simulink model "Demo_TrafficFlow_cs"  (Simulink 7.6) (continued)
== system Demo_TrafficFlow_cs == (continued)
      mask Display: image(imread('ico_OBU_RF_24_gray.png'))
  RFOBU_10_Tx : Subsystem  Tag=OBUTx  Priority=0  mask(PreScan Sink Block: AntennaT){PortName=RFOBU_10, PortIndex=201, PortWidth=16, TimeStep=1/20}
      mask prompts: PortName "Auto Assigned: Port Name [-]"; PortIndex "Auto Assigned: Index [-]"; PortWidth "Auto Assigned: Port Dimensions [-]"; TimeStep "Auto Assigned: Upload Period [s]"
      mask help:
      | Using the Beacon and OBU (On-Board Unit) information between actors and infrastructure can be exchanged.
      |
      | The OBU Transmitter contains the following, user-definable signals:
      |
      | - Vehicle Type		[-]
      | - Spares (1:15)		[-]
      |
      | For more information, please click Help.
      settings: DeleteFcn=PreScanBlockDeleteFcn;
      mask Help: eval('openHelp(487)')
      mask Display: image(imread('ico_OBU_RF_24_gray.png'))
  RFOBU_1_Rx : Subsystem  Tag=OBURx  Priority=3  mask(PreScan Source Block: Antenna Receiver){NrEntitiesOut=2, PortName=RFOBU_1, PortIndex=8, PortWidth=40, TimeStep=1/20}
      mask prompts: NrEntitiesOut "User Specified: # of Visible OBUs"; PortName "Auto Assigned: Port Name [-]"; PortIndex "Auto Assigned: Index [-]"; PortWidth "Auto Assigned: Port Dimensions [-]"; TimeStep "Auto Assigned: Upload Period [s]"
      mask help:
      | Using the Beacon and OBU (On-Board Unit) information between actors and infrastructure can be exchanged.
      |
      | The OBU Reciever receives infrastructure information and user-definable signals.
      |
      | For more information, please click Help.
      settings: DeleteFcn=PreScanBlockDeleteFcn;
      mask Help: eval('openHelp(487)')
      mask Display: image(imread('ico_OBU_RF_24_gray.png'))
  RFOBU_1_Tx : Subsystem  Tag=OBUTx  Priority=0  mask(PreScan Sink Block: AntennaT){PortName=RFOBU_1, PortIndex=15, PortWidth=16, TimeStep=1/20}
      mask prompts: PortName "Auto Assigned: Port Name [-]"; PortIndex "Auto Assigned: Index [-]"; PortWidth "Auto Assigned: Port Dimensions [-]"; TimeStep "Auto Assigned: Upload Period [s]"
      mask help:
      | Using the Beacon and OBU (On-Board Unit) information between actors and infrastructure can be exchanged.
      |
      | The OBU Transmitter contains the following, user-definable signals:
      |
      | - Vehicle Type		[-]
      | - Spares (1:15)		[-]
      |
      | For more information, please click Help.
      settings: DeleteFcn=PreScanBlockDeleteFcn;
      mask Help: eval('openHelp(487)')
      mask Display: image(imread('ico_OBU_RF_24_gray.png'))
  RFOBU_2_Rx : Subsystem  Tag=OBURx  Priority=3  mask(PreScan Source Block: Antenna Receiver){NrEntitiesOut=2, PortName=RFOBU_2, PortIndex=116, PortWidth=40, TimeStep=1/20}
      mask prompts: NrEntitiesOut "User Specified: # of Visible OBUs"; PortName "Auto Assigned: Port Name [-]"; PortIndex "Auto Assigned: Index [-]"; PortWidth "Auto Assigned: Port Dimensions [-]"; TimeStep "Auto Assigned: Upload Period [s]"
      mask help:
      | Using the Beacon and OBU (On-Board Unit) information between actors and infrastructure can be exchanged.
      |
      | The OBU Reciever receives infrastructure information and user-definable signals.
      |
      | For more information, please click Help.
      settings: DeleteFcn=PreScanBlockDeleteFcn;
      mask Help: eval('openHelp(487)')
      mask Display: image(imread('ico_OBU_RF_24_gray.png'))
  RFOBU_2_Tx : Subsystem  Tag=OBUTx  Priority=0  mask(PreScan Sink Block: AntennaT){PortName=RFOBU_2, PortIndex=46, PortWidth=16, TimeStep=1/20}
      mask prompts: PortName "Auto Assigned: Port Name [-]"; PortIndex "Auto Assigned: Index [-]"; PortWidth "Auto Assigned: Port Dimensions [-]"; TimeStep "Auto Assigned: Upload Period [s]"
      mask help:
      | Using the Beacon and OBU (On-Board Unit) information between actors and infrastructure can be exchanged.
      |
      | The OBU Transmitter contains the following, user-definable signals:
      |
      | - Vehicle Type		[-]
      | - Spares (1:15)		[-]
      |
      | For more information, please click Help.
      settings: DeleteFcn=PreScanBlockDeleteFcn;
      mask Help: eval('openHelp(487)')
      mask Display: image(imread('ico_OBU_RF_24_gray.png'))
  RFOBU_3_Rx : Subsystem  Tag=OBURx  Priority=3  mask(PreScan Source Block: Antenna Receiver){NrEntitiesOut=2, PortName=RFOBU_3, PortIndex=224, PortWidth=40, TimeStep=1/20}
      mask prompts: NrEntitiesOut "User Specified: # of Visible OBUs"; PortName "Auto Assigned: Port Name [-]"; PortIndex "Auto Assigned: Index [-]"; PortWidth "Auto Assigned: Port Dimensions [-]"; TimeStep "Auto Assigned: Upload Period [s]"
      mask help:
      | Using the Beacon and OBU (On-Board Unit) information between actors and infrastructure can be exchanged.
      |
      | The OBU Reciever receives infrastructure information and user-definable signals.
      |
      | For more information, please click Help.
      settings: DeleteFcn=PreScanBlockDeleteFcn;
      mask Help: eval('openHelp(487)')
      mask Display: image(imread('ico_OBU_RF_24_gray.png'))
  RFOBU_3_Tx : Subsystem  Tag=OBUTx  Priority=0  mask(PreScan Sink Block: AntennaT){PortName=RFOBU_3, PortIndex=77, PortWidth=16, TimeStep=1/20}
      mask prompts: PortName "Auto Assigned: Port Name [-]"; PortIndex "Auto Assigned: Index [-]"; PortWidth "Auto Assigned: Port Dimensions [-]"; TimeStep "Auto Assigned: Upload Period [s]"
      mask help:
      | Using the Beacon and OBU (On-Board Unit) information between actors and infrastructure can be exchanged.
      |
      | The OBU Transmitter contains the following, user-definable signals:
      |
      | - Vehicle Type		[-]
      | - Spares (1:15)		[-]
      |
      | For more information, please click Help.
      settings: DeleteFcn=PreScanBlockDeleteFcn;
      mask Help: eval('openHelp(487)')
      mask Display: image(imread('ico_OBU_RF_24_gray.png'))
  RFOBU_4_Rx : Subsystem  Tag=OBURx  Priority=3  mask(PreScan Source Block: Antenna Receiver){NrEntitiesOut=2, PortName=RFOBU_4, PortIndex=332, PortWidth=40, TimeStep=1/20}
      mask prompts: NrEntitiesOut "User Specified: # of Visible OBUs"; PortName "Auto Assigned: Port Name [-]"; PortIndex "Auto Assigned: Index [-]"; PortWidth "Auto Assigned: Port Dimensions [-]"; TimeStep "Auto Assigned: Upload Period [s]"
      mask help:
      | Using the Beacon and OBU (On-Board Unit) information between actors and infrastructure can be exchanged.
      |
      | The OBU Reciever receives infrastructure information and user-definable signals.
      |
      | For more information, please click Help.
      settings: DeleteFcn=PreScanBlockDeleteFcn;
      mask Help: eval('openHelp(487)')
      mask Display: image(imread('ico_OBU_RF_24_gray.png'))
  RFOBU_4_Tx : Subsystem  Tag=OBUTx  Priority=0  mask(PreScan Sink Block: AntennaT){PortName=RFOBU_4, PortIndex=108, PortWidth=16, TimeStep=1/20}
      mask prompts: PortName "Auto Assigned: Port Name [-]"; PortIndex "Auto Assigned: Index [-]"; PortWidth "Auto Assigned: Port Dimensions [-]"; TimeStep "Auto Assigned: Upload Period [s]"
      mask help:
      | Using the Beacon and OBU (On-Board Unit) information between actors and infrastructure can be exchanged.
      |
      | The OBU Transmitter contains the following, user-definable signals:
      |
      | - Vehicle Type		[-]
      | - Spares (1:15)		[-]
      |
      | For more information, please click Help.
      settings: DeleteFcn=PreScanBlockDeleteFcn;
      mask Help: eval('openHelp(487)')
      mask Display: image(imread('ico_OBU_RF_24_gray.png'))
  RFOBU_5_Rx : Subsystem  Tag=OBURx  Priority=3  mask(PreScan Source Block: Antenna Receiver){NrEntitiesOut=2, PortName=RFOBU_5, PortIndex=440, PortWidth=40, TimeStep=1/20}
      mask prompts: NrEntitiesOut "User Specified: # of Visible OBUs"; PortName "Auto Assigned: Port Name [-]"; PortIndex "Auto Assigned: Index [-]"; PortWidth "Auto Assigned: Port Dimensions [-]"; TimeStep "Auto Assigned: Upload Period [s]"
      mask help:
      | Using the Beacon and OBU (On-Board Unit) information between actors and infrastructure can be exchanged.
      |
      | The OBU Reciever receives infrastructure information and user-definable signals.
      |
      | For more information, please click Help.
      settings: DeleteFcn=PreScanBlockDeleteFcn;
      mask Help: eval('openHelp(487)')
      mask Display: image(imread('ico_OBU_RF_24_gray.png'))
  RFOBU_5_Tx : Subsystem  Tag=OBUTx  Priority=0  mask(PreScan Sink Block: AntennaT){PortName=RFOBU_5, PortIndex=139, PortWidth=16, TimeStep=1/20}
      mask prompts: PortName "Auto Assigned: Port Name [-]"; PortIndex "Auto Assigned: Index [-]"; PortWidth "Auto Assigned: Port Dimensions [-]"; TimeStep "Auto Assigned: Upload Period [s]"
      mask help:
      | Using the Beacon and OBU (On-Board Unit) information between actors and infrastructure can be exchanged.
      |
      | The OBU Transmitter contains the following, user-definable signals:
      |
      | - Vehicle Type		[-]
      | - Spares (1:15)		[-]
      |
      | For more information, please click Help.
      settings: DeleteFcn=PreScanBlockDeleteFcn;
      mask Help: eval('openHelp(487)')
      mask Display: image(imread('ico_OBU_RF_24_gray.png'))
  RFOBU_6_Rx : Subsystem  Tag=OBURx  Priority=3  mask(PreScan Source Block: Antenna Receiver){NrEntitiesOut=2, PortName=RFOBU_6, PortIndex=548, PortWidth=40, TimeStep=1/20}
      mask prompts: NrEntitiesOut "User Specified: # of Visible OBUs"; PortName "Auto Assigned: Port Name [-]"; PortIndex "Auto Assigned: Index [-]"; PortWidth "Auto Assigned: Port Dimensions [-]"; TimeStep "Auto Assigned: Upload Period [s]"
      mask help:
      | Using the Beacon and OBU (On-Board Unit) information between actors and infrastructure can be exchanged.
      |
      | The OBU Reciever receives infrastructure information and user-definable signals.
      |
      | For more information, please click Help.
      settings: DeleteFcn=PreScanBlockDeleteFcn;
      mask Help: eval('openHelp(487)')
      mask Display: image(imread('ico_OBU_RF_24_gray.png'))
  RFOBU_6_Tx : Subsystem  Tag=OBUTx  Priority=0  mask(PreScan Sink Block: AntennaT){PortName=RFOBU_6, PortIndex=170, PortWidth=16, TimeStep=1/20}
      mask prompts: PortName "Auto Assigned: Port Name [-]"; PortIndex "Auto Assigned: Index [-]"; PortWidth "Auto Assigned: Port Dimensions [-]"; TimeStep "Auto Assigned: Upload Period [s]"
      mask help:
      | Using the Beacon and OBU (On-Board Unit) information between actors and infrastructure can be exchanged.
      |
      | The OBU Transmitter contains the following, user-definable signals:
      |
      | - Vehicle Type		[-]
      | - Spares (1:15)		[-]
      |
      | For more information, please click Help.
      settings: DeleteFcn=PreScanBlockDeleteFcn;
      mask Help: eval('openHelp(487)')
      mask Display: image(imread('ico_OBU_RF_24_gray.png'))
  RFOBU_7_Rx : Subsystem  Tag=OBURx  Priority=3  mask(PreScan Source Block: Antenna Receiver){NrEntitiesOut=2, PortName=RFOBU_7, PortIndex=764, PortWidth=40, TimeStep=1/20}
      mask prompts: NrEntitiesOut "User Specified: # of Visible OBUs"; PortName "Auto Assigned: Port Name [-]"; PortIndex "Auto Assigned: Index [-]"; PortWidth "Auto Assigned: Port Dimensions [-]"; TimeStep "Auto Assigned: Upload Period [s]"
      mask help:
      | Using the Beacon and OBU (On-Board Unit) information between actors and infrastructure can be exchanged.
      |
      | The OBU Reciever receives infrastructure information and user-definable signals.
      |
      | For more information, please click Help.
      settings: DeleteFcn=PreScanBlockDeleteFcn;
      mask Help: eval('openHelp(487)')
      mask Display: image(imread('ico_OBU_RF_24_gray.png'))
  RFOBU_7_Tx : Subsystem  Tag=OBUTx  Priority=0  mask(PreScan Sink Block: AntennaT){PortName=RFOBU_7, PortIndex=232, PortWidth=16, TimeStep=1/20}
      mask prompts: PortName "Auto Assigned: Port Name [-]"; PortIndex "Auto Assigned: Index [-]"; PortWidth "Auto Assigned: Port Dimensions [-]"; TimeStep "Auto Assigned: Upload Period [s]"
      mask help:
      | Using the Beacon and OBU (On-Board Unit) information between actors and infrastructure can be exchanged.
      |
      | The OBU Transmitter contains the following, user-definable signals:
      |
      | - Vehicle Type		[-]
      | - Spares (1:15)		[-]
      |
      | For more information, please click Help.
      settings: DeleteFcn=PreScanBlockDeleteFcn;
      mask Help: eval('openHelp(487)')
      mask Display: image(imread('ico_OBU_RF_24_gray.png'))
  SELF_Audi_A8_1 : Subsystem  Tag=SELF  Priority=3  mask(PreScan Source Block: SELF){PortName=SELF_Audi_A8_1, PortIndex=0, PortWidth=8, TimeStep=1/20}
      mask prompts: PortName "Auto-Assigned: Port Name [-]"; PortIndex "Auto-Assigned: Port Index [-]"; PortWidth "Auto-Assigned: Port Dimensions"; TimeStep "Auto-Assigned: Upload Period [s]"
      mask help:
      | The SELF port shows (state) information of the actor itself. The outputs are position in the experiment's axis system and the GPS coordinates, and the object's absolute velocity and compass heading.
      |
      | For more information, please click Help.
      settings: DeleteFcn=PreScanBlockDeleteFcn;
      mask Help: eval('openHelp(462)')
      mask Display: image(imread('GPS.png'))
  SELF_BMW_X5_1 : Subsystem  Tag=SELF  Priority=3  mask(PreScan Source Block: SELF){PortName=SELF_BMW_X5_1, PortIndex=108, PortWidth=8, TimeStep=1/20}
      mask prompts: PortName "Auto-Assigned: Port Name [-]"; PortIndex "Auto-Assigned: Port Index [-]"; PortWidth "Auto-Assigned: Port Dimensions"; TimeStep "Auto-Assigned: Upload Period [s]"
      mask help:
      | The SELF port shows (state) information of the actor itself. The outputs are position in the experiment's axis system and the GPS coordinates, and the object's absolute velocity and compass heading.
      |
      | For more information, please click Help.
      settings: DeleteFcn=PreScanBlockDeleteFcn;
      mask Help: eval('openHelp(462)')
      mask Display: image(imread('GPS.png'))
  SELF_Citroen_C3_1 : Subsystem  Tag=SELF  Priority=3  mask(PreScan Source Block: SELF){PortName=SELF_Citroen_C3_1, PortIndex=216, PortWidth=8, TimeStep=1/20}
      mask prompts: PortName "Auto-Assigned: Port Name [-]"; PortIndex "Auto-Assigned: Port Index [-]"; PortWidth "Auto-Assigned: Port Dimensions"; TimeStep "Auto-Assigned: Upload Period [s]"
      mask help:
      | The SELF port shows (state) information of the actor itself. The outputs are position in the experiment's axis system and the GPS coordinates, and the object's absolute velocity and compass heading.
      |
      | For more information, please click Help.
      settings: DeleteFcn=PreScanBlockDeleteFcn;
      mask Help: eval('openHelp(462)')
      mask Display: image(imread('GPS.png'))
  SELF_Mazda_RX8_1 : Subsystem  Tag=SELF  Priority=3  mask(PreScan Source Block: SELF){PortName=SELF_Mazda_RX8_1, PortIndex=324, PortWidth=8, TimeStep=1/20}
      mask prompts: PortName "Auto-Assigned: Port Name [-]"; PortIndex "Auto-Assigned: Port Index [-]"; PortWidth "Auto-Assigned: Port Dimensions"; TimeStep "Auto-Assigned: Upload Period [s]"
      mask help:
      | The SELF port shows (state) information of the actor itself. The outputs are position in the experiment's axis system and the GPS coordinates, and the object's absolute velocity and compass heading.
      |
      | For more information, please click Help.
      settings: DeleteFcn=PreScanBlockDeleteFcn;
      mask Help: eval('openHelp(462)')
      mask Display: image(imread('GPS.png'))
  SELF_Motorcyclist_1 : Subsystem  Tag=SELF  Priority=3  mask(PreScan Source Block: SELF){PortName=SELF_Motorcyclist_1, PortIndex=432, PortWidth=8, TimeStep=1/20}
      mask prompts: PortName "Auto-Assigned: Port Name [-]"; PortIndex "Auto-Assigned: Port Index [-]"; PortWidth "Auto-Assigned: Port Dimensions"; TimeStep "Auto-Assigned: Upload Period [s]"
      mask help:
      | The SELF port shows (state) information of the actor itself. The outputs are position in the experiment's axis system and the GPS coordinates, and the object's absolute velocity and compass heading.
      |
      | For more information, please click Help.
      settings: DeleteFcn=PreScanBlockDeleteFcn;
      mask Help: eval('openHelp(462)')
      mask Display: image(imread('GPS.png'))
  SELF_Nissan_Cabstar_1 : Subsystem  Tag=SELF  Priority=3  mask(PreScan Source Block: SELF){PortName=SELF_Nissan_Cabstar_1, PortIndex=540, PortWidth=8, TimeStep=1/20}
      mask prompts: PortName "Auto-Assigned: Port Name [-]"; PortIndex "Auto-Assigned: Port Index [-]"; PortWidth "Auto-Assigned: Port Dimensions"; TimeStep "Auto-Assigned: Upload Period [s]"
      mask help:
      | The SELF port shows (state) information of the actor itself. The outputs are position in the experiment's axis system and the GPS coordinates, and the object's absolute velocity and compass heading.
      |
      | For more information, please click Help.
      settings: DeleteFcn=PreScanBlockDeleteFcn;
      mask Help: eval('openHelp(462)')
      mask Display: image(imread('GPS.png'))
  SELF_Toyota_Previa_1 : Subsystem  Tag=SELF  Priority=3  mask(PreScan Source Block: SELF){PortName=SELF_Toyota_Previa_1, PortIndex=756, PortWidth=8, TimeStep=1/20}
      mask prompts: PortName "Auto-Assigned: Port Name [-]"; PortIndex "Auto-Assigned: Port Index [-]"; PortWidth "Auto-Assigned: Port Dimensions"; TimeStep "Auto-Assigned: Upload Period [s]"
      mask help:
      | The SELF port shows (state) information of the actor itself. The outputs are position in the experiment's axis system and the GPS coordinates, and the object's absolute velocity and compass heading.
      |
      | For more information, please click Help.
      settings: DeleteFcn=PreScanBlockDeleteFcn;
      mask Help: eval('openHelp(462)')
      mask Display: image(imread('GPS.png'))
  SELF_Toyota_Yaris_1 : Subsystem  Tag=SELF  Priority=3  mask(PreScan Source Block: SELF){PortName=SELF_Toyota_Yaris_1, PortIndex=648, PortWidth=8, TimeStep=1/20}
      mask prompts: PortName "Auto-Assigned: Port Name [-]"; PortIndex "Auto-Assigned: Port Index [-]"; PortWidth "Auto-Assigned: Port Dimensions"; TimeStep "Auto-Assigned: Upload Period [s]"
      mask help:
      | The SELF port shows (state) information of the actor itself. The outputs are position in the experiment's axis system and the GPS coordinates, and the object's absolute velocity and compass heading.
      |
      | For more information, please click Help.
      settings: DeleteFcn=PreScanBlockDeleteFcn;
      mask Help: eval('openHelp(462)')
      mask Display: image(imread('GPS.png'))
  STATE_Audi_A8_1 : Subsystem  Tag=State  Priority=0  mask(PreScan Sink Block: State){x0=0, y0=-3.5, z0=0.55, Roll0=0, Pitch0=0, Yaw0=0, TimeStep=1/20}
      mask prompts: x0 "Ini x-position VEHICLE wrt GLOBAL axis system [m]"; y0 "Ini y-position VEHICLE wrt GLOBAL axis system [m]"; z0 "Ini z-position VEHICLE wrt GLOBAL axis system [m]"; Roll0 "Ini roll-angle BODY wrt VEHICLE axis system [deg]"; Pitch0 "Ini pitch-angle BODY wrt VEHICLE axis system [deg]"; Yaw0 "Ini yaw-angle VEHICLE wrt GLOBAL axis system [deg]"; TimeStep "Time Step [s]"
      mask help:
      | The Actor State contains a vector signal of size (9x1), containing the following signals:
      |
      | -  Position                  (x,y,z)                                     [m]
      | -  Velocity                  (vx,vy,vz)                               [m/s]
      | -  Angles                    (Roll, Pitch, Yaw)                   [rad]
      |
      | The Actor state is defined in the global axis system.
      | The state is superponed on the Initial position defined in the GUI.
      |
      | For more information, please click Help.
      settings: DeleteFcn=PreScanBlockDeleteFcn;
      mask Help: eval('openHelp(222)')
      mask Display: image(imread('State.png'))
  STATE_BMW_X5_1 : Subsystem  Tag=State  Priority=0  mask(PreScan Sink Block: State){x0=200, y0=3.5, z0=0.69, Roll0=0, Pitch0=0, Yaw0=180.000412, TimeStep=1/20}
      mask prompts: x0 "Ini x-position VEHICLE wrt GLOBAL axis system [m]"; y0 "Ini y-position VEHICLE wrt GLOBAL axis system [m]"; z0 "Ini z-position VEHICLE wrt GLOBAL axis system [m]"; Roll0 "Ini roll-angle BODY wrt VEHICLE axis system [deg]"; Pitch0 "Ini pitch-angle BODY wrt VEHICLE axis system [deg]"; Yaw0 "Ini yaw-angle VEHICLE wrt GLOBAL axis system [deg]"; TimeStep "Time Step [s]"
      mask help:
      | The Actor State contains a vector signal of size (9x1), containing the following signals:
      |
      | -  Position                  (x,y,z)                                     [m]
      | -  Velocity                  (vx,vy,vz)                               [m/s]
      | -  Angles                    (Roll, Pitch, Yaw)                   [rad]
      |
      | The Actor state is defined in the global axis system.
      | The state is superponed on the Initial position defined in the GUI.
      |
      | For more information, please click Help.
      settings: DeleteFcn=PreScanBlockDeleteFcn;
      mask Help: eval('openHelp(222)')
      mask Display: image(imread('State.png'))
  STATE_Citroen_C3_1 : Subsystem  Tag=State  Priority=0  mask(PreScan Sink Block: State){x0=0, y0=-3.5, z0=0.58, Roll0=0, Pitch0=0, Yaw0=0, TimeStep=1/20}
      mask prompts: x0 "Ini x-position VEHICLE wrt GLOBAL axis system [m]"; y0 "Ini y-position VEHICLE wrt GLOBAL axis system [m]"; z0 "Ini z-position VEHICLE wrt GLOBAL axis system [m]"; Roll0 "Ini roll-angle BODY wrt VEHICLE axis system [deg]"; Pitch0 "Ini pitch-angle BODY wrt VEHICLE axis system [deg]"; Yaw0 "Ini yaw-angle VEHICLE wrt GLOBAL axis system [deg]"; TimeStep "Time Step [s]"
      mask help:
      | The Actor State contains a vector signal of size (9x1), containing the following signals:
      |
      | -  Position                  (x,y,z)                                     [m]
      | -  Velocity                  (vx,vy,vz)                               [m/s]
      | -  Angles                    (Roll, Pitch, Yaw)                   [rad]
      |
      | The Actor state is defined in the global axis system.
      | The state is superponed on the Initial position defined in the GUI.
      |
      | For more information, please click Help.
      settings: DeleteFcn=PreScanBlockDeleteFcn;
      mask Help: eval('openHelp(222)')
      mask Display: image(imread('State.png'))
  STATE_Mazda_RX8_1 : Subsystem  Tag=State  Priority=0  mask(PreScan Sink Block: State){x0=0, y0=-3.5, z0=0.51, Roll0=0, Pitch0=0, Yaw0=0, TimeStep=1/20}
      mask prompts: x0 "Ini x-position VEHICLE wrt GLOBAL axis system [m]"; y0 "Ini y-position VEHICLE wrt GLOBAL axis system [m]"; z0 "Ini z-position VEHICLE wrt GLOBAL axis system [m]"; Roll0 "Ini roll-angle BODY wrt VEHICLE axis system [deg]"; Pitch0 "Ini pitch-angle BODY wrt VEHICLE axis system [deg]"; Yaw0 "Ini yaw-angle VEHICLE wrt GLOBAL axis system [deg]"; TimeStep "Time Step [s]"
      mask help:
      | The Actor State contains a vector signal of size (9x1), containing the following signals:
      |
      | -  Position                  (x,y,z)                                     [m]
      | -  Velocity                  (vx,vy,vz)                               [m/s]
      | -  Angles                    (Roll, Pitch, Yaw)                   [rad]
      |
      | The Actor state is defined in the global axis system.
      | The state is superponed on the Initial position defined in the GUI.
      |
      | For more information, please click Help.
      settings: DeleteFcn=PreScanBlockDeleteFcn;
      mask Help: eval('openHelp(222)')
      mask Display: image(imread('State.png'))
  STATE_Motorcyclist_1 : Subsystem  Tag=State  Priority=0  mask(PreScan Sink Block: State){x0=0, y0=-3.5, z0=0.594, Roll0=0, Pitch0=0, Yaw0=0, TimeStep=1/20}
      mask prompts: x0 "Ini x-position VEHICLE wrt GLOBAL axis system [m]"; y0 "Ini y-position VEHICLE wrt GLOBAL axis system [m]"; z0 "Ini z-position VEHICLE wrt GLOBAL axis system [m]"; Roll0 "Ini roll-angle BODY wrt VEHICLE axis system [deg]"; Pitch0 "Ini pitch-angle BODY wrt VEHICLE axis system [deg]"; Yaw0 "Ini yaw-angle VEHICLE wrt GLOBAL axis system [deg]"; TimeStep "Time Step [s]"
      mask help:
      | The Actor State contains a vector signal of size (9x1), containing the following signals:
      |
      | -  Position                  (x,y,z)                                     [m]
      | -  Velocity                  (vx,vy,vz)                               [m/s]
      | -  Angles                    (Roll, Pitch, Yaw)                   [rad]
      |
      | The Actor state is defined in the global axis system.
      | The state is superponed on the Initial position defined in the GUI.
      |
      | For more information, please click Help.
      settings: DeleteFcn=PreScanBlockDeleteFcn;
      mask Help: eval('openHelp(222)')
      mask Display: image(imread('State.png'))
  STATE_Nissan_Cabstar_1 : Subsystem  Tag=State  Priority=0  mask(PreScan Sink Block: State){x0=200, y0=3.5, z0=0.88, Roll0=0, Pitch0=0, Yaw0=180.000412, TimeStep=1/20}
      mask prompts: x0 "Ini x-position VEHICLE wrt GLOBAL axis system [m]"; y0 "Ini y-position VEHICLE wrt GLOBAL axis system [m]"; z0 "Ini z-position VEHICLE wrt GLOBAL axis system [m]"; Roll0 "Ini roll-angle BODY wrt VEHICLE axis system [deg]"; Pitch0 "Ini pitch-angle BODY wrt VEHICLE axis system [deg]"; Yaw0 "Ini yaw-angle VEHICLE wrt GLOBAL axis system [deg]"; TimeStep "Time Step [s]"
      mask help:
      | The Actor State contains a vector signal of size (9x1), containing the following signals:
      |
      | -  Position                  (x,y,z)                                     [m]
      | -  Velocity                  (vx,vy,vz)                               [m/s]
      | -  Angles                    (Roll, Pitch, Yaw)                   [rad]
      |
      | The Actor state is defined in the global axis system.
      | The state is superponed on the Initial position defined in the GUI.
      |
      | For more information, please click Help.
      settings: DeleteFcn=PreScanBlockDeleteFcn;
      mask Help: eval('openHelp(222)')
      mask Display: image(imread('State.png'))
  STATE_Toyota_Previa_1 : Subsystem  Tag=State  Priority=0  mask(PreScan Sink Block: State){x0=200, y0=3.5, z0=0.71, Roll0=0, Pitch0=0, Yaw0=180.000412, TimeStep=1/20}
      mask prompts: x0 "Ini x-position VEHICLE wrt GLOBAL axis system [m]"; y0 "Ini y-position VEHICLE wrt GLOBAL axis system [m]"; z0 "Ini z-position VEHICLE wrt GLOBAL axis system [m]"; Roll0 "Ini roll-angle BODY wrt VEHICLE axis system [deg]"; Pitch0 "Ini pitch-angle BODY wrt VEHICLE axis system [deg]"; Yaw0 "Ini yaw-angle VEHICLE wrt GLOBAL axis system [deg]"; TimeStep "Time Step [s]"
      mask help:
      | The Actor State contains a vector signal of size (9x1), containing the following signals:
      |
      | -  Position                  (x,y,z)                                     [m]
      | -  Velocity                  (vx,vy,vz)                               [m/s]
      | -  Angles                    (Roll, Pitch, Yaw)                   [rad]
      |
      | The Actor state is defined in the global axis system.
      | The state is superponed on the Initial position defined in the GUI.
      |
      | For more information, please click Help.
      settings: DeleteFcn=PreScanBlockDeleteFcn;
      mask Help: eval('openHelp(222)')
      mask Display: image(imread('State.png'))
  STATE_Toyota_Yaris_1 : Subsystem  Tag=State  Priority=0  mask(PreScan Sink Block: State){x0=200, y0=3.5, z0=0.57, Roll0=0, Pitch0=0, Yaw0=180.000412, TimeStep=1/20}
      mask prompts: x0 "Ini x-position VEHICLE wrt GLOBAL axis system [m]"; y0 "Ini y-position VEHICLE wrt GLOBAL axis system [m]"; z0 "Ini z-position VEHICLE wrt GLOBAL axis system [m]"; Roll0 "Ini roll-angle BODY wrt VEHICLE axis system [deg]"; Pitch0 "Ini pitch-angle BODY wrt VEHICLE axis system [deg]"; Yaw0 "Ini yaw-angle VEHICLE wrt GLOBAL axis system [deg]"; TimeStep "Time Step [s]"
      mask help:
      | The Actor State contains a vector signal of size (9x1), containing the following signals:
      |
      | -  Position                  (x,y,z)                                     [m]
      | -  Velocity                  (vx,vy,vz)                               [m/s]
      | -  Angles                    (Roll, Pitch, Yaw)                   [rad]
      |
      | The Actor state is defined in the global axis system.
      | The state is superponed on the Initial position defined in the GUI.
      |
      | For more information, please click Help.
      settings: DeleteFcn=PreScanBlockDeleteFcn;
      mask Help: eval('openHelp(222)')
      mask Display: image(imread('State.png'))
  SetActuators : Subsystem  Tag=SetActuators  Priority=1  mask(PreScan Simulation Core Block){TimeStep=1/20, NrOfActuators=2338}
      mask prompts: TimeStep "Timestep [s]"; NrOfActuators "Number of actuator values"
      mask help:
      | Please do not remove this block from the PreScan compilation sheet,
      | as it contains essential configuration parameters for a PreScan simulation.
      settings: DeleteFcn=PreScanBlockDeleteFcn;
  SimCoreConfiguration : M-S-Function  Tag=SimCoreConfiguration  Description=A0A32BEFBA57BA318B3979EA0A2895C82458F1CF0CBB3A6E70346316D9276FAC  FunctionName=SimCoreBlock  Parameters=ExperimentXML PortsXML SimulationCore HostName CheckStart EnableLog RfR EnableGPUAccel ShowComputationTime  mask(PreScan Simulation Core Configurator){SimulationCore=NUTS, HostName=0, CheckStart=0, prescanversion='7.4.0', EnableLog=off, RfR=0, EnableGPUAccel=off, ShowComputationTime=off}
      mask prompts: SimulationCore "Simulation Core"; HostName "Simulation Host"; CheckStart "Show START Dialog"; prescanversion "PreScan Version"; EnableLog "Create TA Log"; RfR "Record for Replay"; EnableGPUAccel "Enable GPU Acceleration"; ShowComputationTime "Show ComputationTime Scope"
      mask help:
      | Please do not remove this block from the PreScan compilation sheet,
      | as it contains essential configuration parameter for a PreScan simulation.
      mask init:
      | model = bdroot;
      | ExperimentXML = [model(1:end-3) '.pex'];
      | PortsXML = [model '_ports.pcd'];
      settings: DeleteFcn=PreScanBlockDeleteFcn;  ModelCloseFcn=assignin('base',['model_closed_' bdroot],true);
      mask Help: eval('openHelp(812)')
      mask Display: image(imread('prescanlogo.ico'))
  SimCoreInfo : Subsystem  Tag=SimCoreInfo
  Toyota_Previa_1 : Subsystem  Tag=WorldObjectSubSystem  InitFcn=UniqueId = '59';
      mask Display: image(imread('Toyota_Previa.jpg'));color('white');text(0,0,' ID=21\n')
  Toyota_Yaris_1 : Subsystem  Tag=WorldObjectSubSystem  InitFcn=UniqueId = '57';
      mask Display: image(imread('Toyota_Yaris.jpg'));color('white');text(0,0,' ID=20\n')
  Tripod_1 : Subsystem  Tag=WorldObjectSubSystem  InitFcn=UniqueId = '26';
      mask Display: image(imread('SensorMountPole.jpg'));color('white');text(0,0,' ID=12\n')
  Tripod_2 : Subsystem  Tag=WorldObjectSubSystem  InitFcn=UniqueId = '28';
      mask Display: image(imread('SensorMountPole.jpg'));color('white');text(0,0,' ID=14\n')
  VISUMatrixSignLights_HighwayOverhang_2 : Subsystem  Tag=VisuMatrixSignLights  Priority=0
      settings: DeleteFcn=PreScanBlockDeleteFcn;
      mask Type: PreScan Sink Block: VisuMatrixSign
      mask Description:
      | The VisuMatrixSign block provides a way to adapt the texture of the plates connected to an overhang, based on Resource IDs of the texture files.
      | 
      | For more information, please click Help.
      mask Help: eval('openHelp()')
      mask Display: image(imread('HighwaySpeedMatrix.png'))
  VISUMatrixSign_HighwayOverhang_2 : Subsystem  Tag=VisuMatrixSign  Priority=0
      settings: DeleteFcn=PreScanBlockDeleteFcn;
      mask Type: PreScan Sink Block: VisuMatrixSign
      mask Description:
      | The VisuMatrixSign block provides a way to adapt the texture of the plates connected to an overhang, based on Resource IDs of the texture files.
      | 
      | For more information, please click Help.
      mask Help: eval('openHelp()')
      mask Display: image(imread('HighwaySpeedMatrix.png'))
  wires:
  Audi_A8_1/1(STATE_Audi_A8_1) -> STATE_Audi_A8_1/1(State)
  SELF_Audi_A8_1/1(U) -> Audi_A8_1/1(SELF_Audi_A8_1)
  RFOBU_1_Rx/1(U) -> Audi_A8_1/2(RFOBU_1_Rx)
  AIR_1/1 -> Audi_A8_1/3(AIR_1)
  Audi_A8_1/2(RFOBU_1_Tx) -> RFOBU_1_Tx/1(Y)
  BMW_X5_1/1(STATE_BMW_X5_1) -> STATE_BMW_X5_1/1(State)
  SELF_BMW_X5_1/1(U) -> BMW_X5_1/1(SELF_BMW_X5_1)
  RFOBU_2_Rx/1(U) -> BMW_X5_1/2(RFOBU_2_Rx)
  AIR_2/1 -> BMW_X5_1/3(AIR_2)
  BMW_X5_1/2(RFOBU_2_Tx) -> RFOBU_2_Tx/1(Y)
  Citroen_C3_1/1(STATE_Citroen_C3_1) -> STATE_Citroen_C3_1/1(State)
  SELF_Citroen_C3_1/1(U) -> Citroen_C3_1/1(SELF_Citroen_C3_1)
  RFOBU_3_Rx/1(U) -> Citroen_C3_1/2(RFOBU_3_Rx)
  AIR_3/1 -> Citroen_C3_1/3(AIR_3)
  Citroen_C3_1/2(RFOBU_3_Tx) -> RFOBU_3_Tx/1(Y)
  Mazda_RX8_1/1(STATE_Mazda_RX8_1) -> STATE_Mazda_RX8_1/1(State)
  SELF_Mazda_RX8_1/1(U) -> Mazda_RX8_1/1(SELF_Mazda_RX8_1)
  RFOBU_4_Rx/1(U) -> Mazda_RX8_1/2(RFOBU_4_Rx)
  AIR_4/1 -> Mazda_RX8_1/3(AIR_4)
  Mazda_RX8_1/2(RFOBU_4_Tx) -> RFOBU_4_Tx/1(Y)
  Motorcyclist_1/1(STATE_Motorcyclist_1) -> STATE_Motorcyclist_1/1(State)
  SELF_Motorcyclist_1/1(U) -> Motorcyclist_1/1(SELF_Motorcyclist_1)
  RFOBU_5_Rx/1(U) -> Motorcyclist_1/2(RFOBU_5_Rx)
  AIR_5/1 -> Motorcyclist_1/3(AIR_5)
  Motorcyclist_1/2(RFOBU_5_Tx) -> RFOBU_5_Tx/1(Y)
  Nissan_Cabstar_1/1(STATE_Nissan_Cabstar_1) -> STATE_Nissan_Cabstar_1/1(State)
  SELF_Nissan_Cabstar_1/1(U) -> Nissan_Cabstar_1/1(SELF_Nissan_Cabstar_1)
  RFOBU_6_Rx/1(U) -> Nissan_Cabstar_1/2(RFOBU_6_Rx)
  AIR_6/1 -> Nissan_Cabstar_1/3(AIR_6)
  Nissan_Cabstar_1/2(RFOBU_6_Tx) -> RFOBU_6_Tx/1(Y)
  Toyota_Yaris_1/1(STATE_Toyota_Yaris_1) -> STATE_Toyota_Yaris_1/1(State)
  SELF_Toyota_Yaris_1/1(U) -> Toyota_Yaris_1/1(SELF_Toyota_Yaris_1)
  RFOBU_10_Rx/1(U) -> Toyota_Yaris_1/2(RFOBU_10_Rx)
  AIR_7/1 -> Toyota_Yaris_1/3(AIR_7)
  Toyota_Yaris_1/2(RFOBU_10_Tx) -> RFOBU_10_Tx/1(Y)
  Toyota_Previa_1/1(STATE_Toyota_Previa_1) -> STATE_Toyota_Previa_1/1(State)
  SELF_Toyota_Previa_1/1(U) -> Toyota_Previa_1/1(SELF_Toyota_Previa_1)
  RFOBU_7_Rx/1(U) -> Toyota_Previa_1/2(RFOBU_7_Rx)
  AIR_8/1 -> Toyota_Previa_1/3(AIR_8)
  Toyota_Previa_1/2(RFOBU_7_Tx) -> RFOBU_7_Tx/1(Y)
  RFBeacon_1_Rx/1(U) -> HighwayOverhang_1/1(RFBeacon_1_Rx)
  Antenna_Rx_2/1(U) -> HighwayOverhang_1/2(Antenna_Rx_2)
  HighwayOverhang_1/1(Antenna_Tx_2) -> Antenna_Tx_2/1(Y)
  HighwayOverhang_1/2(RFBeacon_1_Tx) -> RFBeacon_1_Tx/1(Y)
  RFBeacon_2_Rx/1(U) -> HighwayOverhang_2/1(RFBeacon_2_Rx)
  Antenna_Rx_3/1(U) -> HighwayOverhang_2/2(Antenna_Rx_3)
  HighwayOverhang_2/1(Antenna_Tx_3) -> Antenna_Tx_3/1(Y)
  HighwayOverhang_2/2(RFBeacon_2_Tx) -> RFBeacon_2_Tx/1(Y)
  HighwayOverhang_2/3(VISUMatrixSign_HighwayOverhang_2) -> VISUMatrixSign_HighwayOverhang_2/1(MatrixSigns)
  HighwayOverhang_2/4(VISUMatrixSignLights_HighwayOverhang_2) -> VISUMatrixSignLights_HighwayOverhang_2/1(MatrixSignLights)
  RFBeacon_3_Rx/1(U) -> HighwayOverhang_3/1(RFBeacon_3_Rx)
  Antenna_Rx_4/1(U) -> HighwayOverhang_3/2(Antenna_Rx_4)
  HighwayOverhang_3/1(Antenna_Tx_4) -> Antenna_Tx_4/1(Y)
  HighwayOverhang_3/2(RFBeacon_3_Tx) -> RFBeacon_3_Tx/1(Y)
  Antenna_Rx_7/1(U) -> Control_Unit/1(Antenna_Rx_7)
  Control_Unit/1(Antenna_Tx_7) -> Antenna_Tx_7/1(Y)
  Antenna_Rx_8/1(U) -> Control_Unit/2(Antenna_Rx_8)
  Control_Unit/2(Antenna_Tx_8) -> Antenna_Tx_8/1(Y)
  Antenna_Rx_9/1(U) -> Control_Unit/3(Antenna_Rx_9)
  Control_Unit/3(Antenna_Tx_9) -> Antenna_Tx_9/1(Y)
  RFBeacon_4_Rx/1(U) -> Tripod_1/1(RFBeacon_4_Rx)
  Tripod_1/1(RFBeacon_4_Tx) -> RFBeacon_4_Tx/1(Y)
  RFBeacon_5_Rx/1(U) -> Tripod_2/1(RFBeacon_5_Rx)
  Tripod_2/1(RFBeacon_5_Tx) -> RFBeacon_5_Tx/1(Y)
  SetActuators/1(dummy) -> GetSensors/1(dummy)
